simulink model "hyd_hyb_2_opt_cont_test_new_1_col_ef_fin_2"  (Simulink 7.7) (continued)
== system hyd_hyb_2_opt_cont_test_new_1_col_ef_fin_2/controller2/optimized controller/SOC upper 0.5/SOC upper 0.6/SOC under 0.7/net_col_e_f_6_7/Layer 2/LW{2,1} == (continued)
      mask Display: e = 0.06;c1 = [120 150 230]/255;c2 = ([120 150 230]+0)/383;c3 = ([120 150 230]+128)/383;patch([0 1 1 0],[0 0 1 1],c1);patch([0 1 1 1-e 1-e 0+e],[0 0 1 1-e 0+e 0+e],c2);patch([0 0 1 1-e 0+e 0+e],[0 1 1 1-e 1-e 0+e],c3);plot([0 1 1 0 0],[0 0 1 1 0]);disp('weights');
  "IW{2,1}(33,:)'" : Constant  Value=[-0.35234469279286512;-0.40468235895147475;-0.18096524442613818;-0.18151288248094333;0.35857480457232321;0.23465283463217285;0.40101555825099472;0.21139093219043778;-0.11912748111615507;0.29342558210195213;-0.15948625047030618;-0.11815965704262263;0.1116227020812456;0.38214609794411863;-0.35421490398026861;-0.27312335423238421;-0.26788573951818151;0.021395386612412425;-0.31654082740969164;-0.083409909154861142;-0.1468782051998643;0.21847941839715651;0.37294860709219418;0.2637687675371283;-0.074718043753328647;0.12745812216454461;-0.056240044727995646;0.22653279906164184;0.32679539257000695;0.33557761404320691;-0.01958919024617814;0.056572444135230458;0.30300666085920641;0.011579914144332524;0.26738390697574438;0.34894240758758227;-0.13804654423913723;0.13383865475945017;-0.21150677078154806;0.090005117551270664]
      mask Display: e = 0.06;c1 = [120 150 230]/255;c2 = ([120 150 230]+0)/383;c3 = ([120 150 230]+128)/383;patch([0 1 1 0],[0 0 1 1],c1);patch([0 1 1 1-e 1-e 0+e],[0 0 1 1-e 0+e 0+e],c2);patch([0 0 1 1-e 0+e 0+e],[0 1 1 1-e 1-e 0+e],c3);plot([0 1 1 0 0],[0 0 1 1 0]);disp('weights');
  "IW{2,1}(34,:)'" : Constant  Value=[0.15074995802727087;0.30864088922738236;0.10562014308619606;0.029979241891492325;-0.15584734818296847;-0.26021048893016635;-0.24865146505391678;0.41604231588507162;0.045896804265341991;-0.08694543014273505;-0.085027594542836857;0.45862186579974429;-0.31361816930303732;-0.12097477912022493;0.28844762834441939;0.066038996178467271;-0.068572896253808427;-0.09175843487790733;0.44634530134350081;0.17315383144725219;-0.29035181630262313;0.34479646540728387;-0.30962740604637629;-0.22996363447586768;-0.22948723755561082;0.31622260509068861;-0.25078044542389749;-0.37447691479609285;-0.35113036697226169;-0.089397504594286262;-0.255187761364293;0.079247556333326974;-0.20079103297549039;-0.074861134771812943;0.27127698682470541;0.38487777094434789;-0.2471333962217872;-0.089882718136514175;-0.20102325084023667;0.23002736531891085]
      mask Display: e = 0.06;c1 = [120 150 230]/255;c2 = ([120 150 230]+0)/383;c3 = ([120 150 230]+128)/383;patch([0 1 1 0],[0 0 1 1],c1);patch([0 1 1 1-e 1-e 0+e],[0 0 1 1-e 0+e 0+e],c2);patch([0 0 1 1-e 0+e 0+e],[0 1 1 1-e 1-e 0+e],c3);plot([0 1 1 0 0],[0 0 1 1 0]);disp('weights');
  "IW{2,1}(35,:)'" : Constant  Value=[-0.085280357024164019;-0.15814604519744255;-0.24621739793110575;0.43143057576690308;-0.42363845727206917;0.08687226022397769;0.21079716523428366;-0.024164079180984198;0.42797572604181494;0.4498416033934976;0.10822528990763226;0.085574245922645745;0.1522570064361313;-0.23438964187238326;0.2505811617273031;0.37801894202352326;-0.24299010034030982;0.019423098657966726;-0.10410706384354135;0.12024117541164461;0.37963413538941615;0.20515543726246629;-0.38789940580890198;0.10317756927625593;-0.36671922248120226;0.10963932782027253;0.074473878145015535;0.25249510438191625;0.022718384464952383;0.089004438179455844;-0.1812967994841308;-0.099778907647907422;-0.00013885403074624433;-0.35509553735099791;-0.02986583737910134;0.26514173132914987;0.41024463729816096;0.31064560212289161;0.22581215848793651;0.084557763920990112]
      mask Display: e = 0.06;c1 = [120 150 230]/255;c2 = ([120 150 230]+0)/383;c3 = ([120 150 230]+128)/383;patch([0 1 1 0],[0 0 1 1],c1);patch([0 1 1 1-e 1-e 0+e],[0 0 1 1-e 0+e 0+e],c2);patch([0 0 1 1-e 0+e 0+e],[0 1 1 1-e 1-e 0+e],c3);plot([0 1 1 0 0],[0 0 1 1 0]);disp('weights');
  "IW{2,1}(36,:)'" : Constant  Value=[0.11187917842212827;-0.15833534714410497;0.21442117909747105;0.18035201202815951;-0.20103443165034554;-0.43676294389703935;-0.24767795813336665;0.42859594714433347;-0.46594462872991904;0.38609488819476268;0.42804363407379792;-0.24835357807569508;0.35487975603085459;0.099382895784186964;0.037702915655006712;0.077359712591388144;-0.042553881876517007;0.22802448851846341;-0.15502215013149115;0.046833480077730839;-0.010626970787021696;-0.15587949850514998;0.10795274546066105;-0.35647469267790022;0.093489866349711181;-0.061913015016581703;0.0069346425814466352;0.23410829073547867;0.082823681928375095;0.084672792381860665;0.063329516746211914;0.22751356665680345;0.26551938770123051;-0.43991509625618636;-0.19202855444821165;0.1000567847980213;0.1197961732104279;-0.32733847522348158;0.36479375386410845;0.2732239191085889]
      mask Display: e = 0.06;c1 = [120 150 230]/255;c2 = ([120 150 230]+0)/383;c3 = ([120 150 230]+128)/383;patch([0 1 1 0],[0 0 1 1],c1);patch([0 1 1 1-e 1-e 0+e],[0 0 1 1-e 0+e 0+e],c2);patch([0 0 1 1-e 0+e 0+e],[0 1 1 1-e 1-e 0+e],c3);plot([0 1 1 0 0],[0 0 1 1 0]);disp('weights');
  "IW{2,1}(37,:)'" : Constant  Value=[0.22542906269712015;-0.23206687387491265;-0.41582441453719304;0.19907958152951435;-0.052390899388030987;-0.21713307410620905;-0.14279836338394636;-0.18034106153095997;0.38195893101400419;-0.20167227233343965;-0.10798221528183477;0.12776729738967238;0.12347559665720846;-0.39361066407783174;0.057989056419332705;-0.084862484010496314;-0.033437615546936063;-0.11558028023985598;0.021886547193143046;-0.34502232466084698;-0.3357414012306229;0.30873569567980058;-0.34857502847280747;-0.25620889903961552;0.14020136652308349;0.20416194799760654;-0.11813950443884963;0.27495061115104813;0.23013740973878341;-0.38843845697959822;-0.23459303861170966;0.29930194635965313;0.24309548875365156;0.256556613125552;0.41238162631707992;0.18772611001470427;-0.076315885440117276;0.012893512983791903;-0.43634903569871925;0.065732915816523241]
      mask Display: e = 0.06;c1 = [120 150 230]/255;c2 = ([120 150 230]+0)/383;c3 = ([120 150 230]+128)/383;patch([0 1 1 0],[0 0 1 1],c1);patch([0 1 1 1-e 1-e 0+e],[0 0 1 1-e 0+e 0+e],c2);patch([0 0 1 1-e 0+e 0+e],[0 1 1 1-e 1-e 0+e],c3);plot([0 1 1 0 0],[0 0 1 1 0]);disp('weights');
  "IW{2,1}(38,:)'" : Constant  Value=[-0.15687637927286577;0.36917633563686009;-0.29144238642137027;-0.28496243830489176;-0.29120265296463177;-0.16720472422586266;-0.32732491418049114;0.012380328221147003;0.25308608987500869;-0.31080168284313453;-0.14115897836824481;0.30823959639828868;0.13435007189910428;-0.27026968452106997;0.33353108706042345;-0.20586162066266542;0.35646268375518114;-0.29786664275900671;-0.037532512390921584;-0.23059109371293018;-0.32874031983206159;-0.065775359524783569;0.31255364238137584;0.13357880972227706;-0.32569441413025574;0.32777707861072408;-0.092206732296571184;0.12182727513782284;0.19694934568800054;-0.29716135192519855;0.12372996132474567;-0.29314142212795796;0.1007453511214564;0.12695639234602313;0.19962832326613919;-0.19600314744227701;-0.15468264750313671;0.27220846671165405;-0.36357404568227414;-0.22248043670160675]
      mask Display: e = 0.06;c1 = [120 150 230]/255;c2 = ([120 150 230]+0)/383;c3 = ([120 150 230]+128)/383;patch([0 1 1 0],[0 0 1 1],c1);patch([0 1 1 1-e 1-e 0+e],[0 0 1 1-e 0+e 0+e],c2);patch([0 0 1 1-e 0+e 0+e],[0 1 1 1-e 1-e 0+e],c3);plot([0 1 1 0 0],[0 0 1 1 0]);disp('weights');
  "IW{2,1}(39,:)'" : Constant  Value=[0.24058796492975254;0.3923243587570851;0.078966515972267892;-0.16394619579342773;-0.30280913050520986;0.3484741459001085;-0.12726912018809577;-0.22962738727879989;-0.04388378471388131;-0.23687844282218948;0.023563965371155793;-0.31482535629267333;-0.39420955887147413;-0.29577827442781379;-0.35103390143920965;0.063402770474746808;0.3284365627069033;0.1595091253598937;0.11070826187861493;-0.044032202526256531;0.36978057342829418;0.35721143459447674;0.16964431808255342;0.020192388082055583;-0.046017910286698605;0.402278478482547;0.11348150264565539;-0.43801214172903946;0.087002884619087589;0.071672830442686686;0.050469899960920474;-0.35116292344382749;-0.037997808720628551;0.14157546914372149;0.026100866768060997;-0.079742117838089863;0.08707088759100097;-0.36495123765462395;-0.35755801475726767;-0.26509645505088503]
      mask Display: e = 0.06;c1 = [120 150 230]/255;c2 = ([120 150 230]+0)/383;c3 = ([120 150 230]+128)/383;patch([0 1 1 0],[0 0 1 1],c1);patch([0 1 1 1-e 1-e 0+e],[0 0 1 1-e 0+e 0+e],c2);patch([0 0 1 1-e 0+e 0+e],[0 1 1 1-e 1-e 0+e],c3);plot([0 1 1 0 0],[0 0 1 1 0]);disp('weights');
  "IW{2,1}(4,:)'" : Constant  Value=[-0.01221140323552884;0.18072786298812832;-0.3140855165908864;-0.12099788973110417;-0.14523488288702574;-0.43700931256781916;0.42561098451762502;-0.21444295716308656;-0.41057632388322274;-0.37893180528552617;-0.031683877853917852;-0.23036116027979819;-0.16473141780181505;-0.35253387674590198;0.341963767625098;0.23912597139523462;0.30909431730506876;-0.36697578035509515;0.24200988103104415;-0.17643789536103216;-0.001019241624209845;0.29537860690834722;0.016094459145938728;-0.037543658468204864;-0.32564370819347827;-0.14949886992390565;0.0054613804968836827;-0.076340588784367183;-0.038168613201275396;0.26431828047945816;0.1920327419058856;-0.20127733354280752;-0.17784322199890351;0.1276306481919468;0.063327827863554592;0.13946038997141527;-0.12252141040949782;0.39836882652049344;-0.39764916157177288;-0.35786507616559005]
      mask Display: e = 0.06;c1 = [120 150 230]/255;c2 = ([120 150 230]+0)/383;c3 = ([120 150 230]+128)/383;patch([0 1 1 0],[0 0 1 1],c1);patch([0 1 1 1-e 1-e 0+e],[0 0 1 1-e 0+e 0+e],c2);patch([0 0 1 1-e 0+e 0+e],[0 1 1 1-e 1-e 0+e],c3);plot([0 1 1 0 0],[0 0 1 1 0]);disp('weights');
  "IW{2,1}(40,:)'" : Constant  Value=[-0.2977786014958943;0.18727182475970403;0.13467798845472928;0.076857101125664309;-0.18584526349064118;0.35512226754925064;-0.15661520913315033;-0.39811458268789479;0.38617345102519257;-0.17197958098024463;-0.34058983039534269;-0.13495905846225914;-0.18481750329056115;-0.08486830956154133;0.32310252648926008;-0.088847963238082611;-0.26900255736207407;0.42295876308626307;-0.16258324878140534;-0.075092546961337936;0.26431985915047473;-0.13978120836778424;-0.44168942945243578;0.14522828817594052;-0.081041133166638649;0.0034634335491892159;-0.084042534105895017;0.41568204161889105;0.31353846767808768;0.32386770769435796;-0.16174740047881567;0.26074364045686615;-0.16612768934716163;0.077983879794636363;0.15478651429146739;-0.35317869634842858;0.33259676584433623;-0.26549581598378302;-0.07896726765929496;-0.26229401937605112]
      mask Display: e = 0.06;c1 = [120 150 230]/255;c2 = ([120 150 230]+0)/383;c3 = ([120 150 230]+128)/383;patch([0 1 1 0],[0 0 1 1],c1);patch([0 1 1 1-e 1-e 0+e],[0 0 1 1-e 0+e 0+e],c2);patch([0 0 1 1-e 0+e 0+e],[0 1 1 1-e 1-e 0+e],c3);plot([0 1 1 0 0],[0 0 1 1 0]);disp('weights');
  "IW{2,1}(41,:)'" : Constant  Value=[0.12374463207755997;-0.28158655207815841;0.095589009979011041;-0.3235745029745532;-0.27310967972902223;0.22733011791120317;0.19831808496259232;0.00057962201351577597;0.071724425828926811;0.19048384458678946;0.36773949834236991;0.064053855217139147;-0.035613463718706634;-0.20216967365801861;-0.28007020467049937;0.24364774186005511;-0.1403404377486597;-0.050967598966734931;-0.16763395213635215;-0.20341622710789936;0.43084514323429318;-0.24361074047060993;-0.28677350264557416;0.14571519093268848;-0.23874680606219301;-0.25250218454533557;0.31533910494837741;0.38945443402948615;-0.13606527960694581;0.062069561913058138;0.088721912161191269;0.047018434001795527;-0.37942370927896968;-0.40725710006095339;-0.33740501650702037;0.2478864625186305;0.28386365132508418;-0.14097524665361313;0.23075320657061499;0.34003083768377967]
      mask Display: e = 0.06;c1 = [120 150 230]/255;c2 = ([120 150 230]+0)/383;c3 = ([120 150 230]+128)/383;patch([0 1 1 0],[0 0 1 1],c1);patch([0 1 1 1-e 1-e 0+e],[0 0 1 1-e 0+e 0+e],c2);patch([0 0 1 1-e 0+e 0+e],[0 1 1 1-e 1-e 0+e],c3);plot([0 1 1 0 0],[0 0 1 1 0]);disp('weights');
  "IW{2,1}(42,:)'" : Constant  Value=[-0.3505777576555813;-0.10999602423138424;0.30553694772179868;-0.10664651929504305;-0.30249080816352231;-0.13003161728713097;0.30683350520583913;0.41317558957586309;-0.30389328175577485;-0.21695178164448795;0.0031775306979481437;-0.012427164986180059;0.061179138449127923;0.23413387880260014;-0.11431185560742893;0.19921615325010597;0.28718734724044997;0.17696705069996821;-0.37706459191558217;-0.078774553000733369;-0.31208712531433175;-0.3569580615326558;0.22140312811492585;0.051341593285318773;0.32120033086946859;-0.030192407869492219;-0.14634065749356878;-0.26831791640789687;0.13749622178982507;-0.18708157332632702;-0.13830824842391906;-0.23059500983019787;-0.44154847323212926;-0.27873882384836446;0.28486587161326749;0.37593153678044644;0.42781288247884747;0.0032857441186048661;0.041166282396126533;-0.12426148756994582]
      mask Display: e = 0.06;c1 = [120 150 230]/255;c2 = ([120 150 230]+0)/383;c3 = ([120 150 230]+128)/383;patch([0 1 1 0],[0 0 1 1],c1);patch([0 1 1 1-e 1-e 0+e],[0 0 1 1-e 0+e 0+e],c2);patch([0 0 1 1-e 0+e 0+e],[0 1 1 1-e 1-e 0+e],c3);plot([0 1 1 0 0],[0 0 1 1 0]);disp('weights');
  "IW{2,1}(43,:)'" : Constant  Value=[-0.28284206118375033;-0.36311546878953466;0.30689587766983428;0.108845991936885;-0.24031228944312771;0.30154934077932222;-0.25412610019440668;-0.01918150170030811;0.28581766315806073;-0.10785434376307283;-0.25949570248524989;0.23479396205927056;-0.35613185960404459;-0.21607664044194339;-0.37283429390225165;0.23790879215982905;0.31868259314811448;-0.17765900646240576;0.30326262719508662;-0.045899657726886041;-0.31470971859168284;0.34019858096204886;-0.055034131947890023;-0.098124487040463532;-0.079524074549746346;0.24640470466577152;0.13875347903159121;-0.37489966207229808;0.2300931495036142;0.25528449654750995;0.12450861327235381;-0.13224675687262377;-0.32954454098584407;0.042449840898941077;0.35561545193798566;0.13425562374375302;-0.10150108311114024;-0.18589732358968489;-0.048415597487845657;-0.31802719225086684]
      mask Display: e = 0.06;c1 = [120 150 230]/255;c2 = ([120 150 230]+0)/383;c3 = ([120 150 230]+128)/383;patch([0 1 1 0],[0 0 1 1],c1);patch([0 1 1 1-e 1-e 0+e],[0 0 1 1-e 0+e 0+e],c2);patch([0 0 1 1-e 0+e 0+e],[0 1 1 1-e 1-e 0+e],c3);plot([0 1 1 0 0],[0 0 1 1 0]);disp('weights');
  "IW{2,1}(44,:)'" : Constant  Value=[0.24186756609886367;0.095626761068682481;0.26654656067729149;0.39643642757555436;-0.10425918489107079;-0.26391489579144017;-0.049893019446727367;-0.36958558815436737;-0.41536315585712119;-0.25940660039384267;0.28266445101991661;-0.37749239778290772;0.087014706296541219;-0.033609775651372818;-0.21665966922491384;0.06825768093279766;-0.40289599529495396;0.37876927109177572;-0.41873452944884437;0.24178274879595246;0.068391117677172486;0.095644761277350762;0.35331142901495649;0.058087080343530051;0.14037668371934481;0.20057470271574948;-0.28969763356565392;0.18991334315756131;0.071977111117728323;0.007847130043674537;0.2429287207714966;-0.24668758835818619;-0.19617478229712637;0.091426183332240937;-0.083169358743805169;-0.10413849932149064;0.27713246312576362;0.078656969478348909;0.36810666213474291;-0.30832420447422881]
      mask Display: e = 0.06;c1 = [120 150 230]/255;c2 = ([120 150 230]+0)/383;c3 = ([120 150 230]+128)/383;patch([0 1 1 0],[0 0 1 1],c1);patch([0 1 1 1-e 1-e 0+e],[0 0 1 1-e 0+e 0+e],c2);patch([0 0 1 1-e 0+e 0+e],[0 1 1 1-e 1-e 0+e],c3);plot([0 1 1 0 0],[0 0 1 1 0]);disp('weights');
  "IW{2,1}(45,:)'" : Constant  Value=[0.15801553328063686;0.2147950848714196;-0.10432151961439522;0.35287803443813565;-0.41349923728397608;0.12549140028135947;0.39922863113523932;-0.03498605999225167;-0.28755280948461548;-0.36499970043289315;-0.20358994128441979;-0.25782078255905444;0.011922789908669391;0.25532328024521378;-0.18723327739464099;0.28325370995158766;-0.25319754558012314;-0.23325080914514792;-0.066741860102844539;0.034476643291110803;0.14136647287229664;-0.27029024634068632;0.27454415669704146;-0.13749499473286073;0.31368285562019016;-0.12419702471524841;0.25200297985223702;0.39190773946821061;0.26454713274680408;-0.065334327629809924;-0.25503977586253629;0.0015780512603695012;0.36547133487036465;-0.42034430951905716;-0.13031134885640877;0.014551092701692156;0.39892420847612808;-0.17406942193360311;0.062344711607968943;-0.15053134249806502]
      mask Display: e = 0.06;c1 = [120 150 230]/255;c2 = ([120 150 230]+0)/383;c3 = ([120 150 230]+128)/383;patch([0 1 1 0],[0 0 1 1],c1);patch([0 1 1 1-e 1-e 0+e],[0 0 1 1-e 0+e 0+e],c2);patch([0 0 1 1-e 0+e 0+e],[0 1 1 1-e 1-e 0+e],c3);plot([0 1 1 0 0],[0 0 1 1 0]);disp('weights');
  "IW{2,1}(46,:)'" : Constant  Value=[-0.25346893568143747;0.11674230260896117;0.21247665319827722;-0.3023226316265718;0.40187215509063173;0.25179388244739503;-0.35482660278569744;-0.41651174379826861;-0.32396559297957056;0.077915869952318945;0.22280449623257417;0.10877322968338277;0.036141294556576831;-0.3485358781664159;0.32141807436619746;0.14854376989108528;0.10812867363128655;-0.26938446979961433;-0.25835872660066272;0.23251585870346667;0.40716747714170437;0.26793414586456421;-0.075165437384607334;0.29601224621827904;0.14197703179086099;-0.013831111239489052;-0.26813860878220697;0.34810237060033167;-0.28500503795060689;0.18951683840777356;-0.24261999984330401;0.10497856057091108;-0.012566490475294125;-0.16805804081586809;0.25540243616744723;0.31061497654270043;-0.27989029998371051;0.29625468641388025;-0.12489425730626975;0.012148501887610281]
      mask Display: e = 0.06;c1 = [120 150 230]/255;c2 = ([120 150 230]+0)/383;c3 = ([120 150 230]+128)/383;patch([0 1 1 0],[0 0 1 1],c1);patch([0 1 1 1-e 1-e 0+e],[0 0 1 1-e 0+e 0+e],c2);patch([0 0 1 1-e 0+e 0+e],[0 1 1 1-e 1-e 0+e],c3);plot([0 1 1 0 0],[0 0 1 1 0]);disp('weights');
  "IW{2,1}(47,:)'" : Constant  Value=[-0.16854743580021067;0.095087222038890029;0.24743230793525181;0.29113750231583885;0.33027710233818858;0.11584491491617656;0.31235286979033411;-0.25808866350084159;-0.22181754755204813;-0.20800748339377717;-0.27377489451822568;-0.18961470743893991;0.33024903069429828;0.09709101495274014;-0.19774033278608544;0.25622034772817109;-0.12761057645318893;-0.28207402884870236;0.32359854685351719;0.19148606877682869;0.1659100818996371;-0.22896322697040691;-0.11690933194391534;0.28206751525415219;0.19309652461392576;-0.32135572428465964;0.34436465561563601;0.25491020099889378;0.039812368894199222;-0.00045147899089443709;0.35801807198273933;-0.44150383595354553;-0.39280237255984529;-0.043673666389801344;-0.0044382786984080392;0.30094944022010744;0.17955990880744432;-0.11371270789555313;0.37299467764138;-0.042167474381799221]
      mask Display: e = 0.06;c1 = [120 150 230]/255;c2 = ([120 150 230]+0)/383;c3 = ([120 150 230]+128)/383;patch([0 1 1 0],[0 0 1 1],c1);patch([0 1 1 1-e 1-e 0+e],[0 0 1 1-e 0+e 0+e],c2);patch([0 0 1 1-e 0+e 0+e],[0 1 1 1-e 1-e 0+e],c3);plot([0 1 1 0 0],[0 0 1 1 0]);disp('weights');
  "IW{2,1}(48,:)'" : Constant  Value=[0.33580983684677068;-0.3557646378436905;-0.2609869867761912;0.41804217770025731;0.023018027558655944;0.30021868143595909;-0.21436857746172339;-0.33228020411141806;0.19401784216186033;0.18821683826027716;-0.29336978856191481;-0.29960260186473381;-0.079237285858170914;-0.10052576060754399;0.42546444450459026;-0.20530312551278571;-0.25493138728255849;-0.15575443563409963;-0.24795824142890097;0.21714853416644914;-0.17629417880485057;0.41521854751890536;-0.025068130748208971;0.078460162700272174;-0.22200641029381338;-0.14732513315524082;0.084248299372936583;-0.066942842273487735;-0.018727673723746188;0.25505570248698373;-0.26857039064822424;-0.16545383905599903;-0.17576286045151351;-0.33753246371969065;0.27704733533396336;0.11682621568904474;-0.34026989373512978;0.022581869684723314;-0.3851876763316629;0.10156330710757971]
      mask Display: e = 0.06;c1 = [120 150 230]/255;c2 = ([120 150 230]+0)/383;c3 = ([120 150 230]+128)/383;patch([0 1 1 0],[0 0 1 1],c1);patch([0 1 1 1-e 1-e 0+e],[0 0 1 1-e 0+e 0+e],c2);patch([0 0 1 1-e 0+e 0+e],[0 1 1 1-e 1-e 0+e],c3);plot([0 1 1 0 0],[0 0 1 1 0]);disp('weights');
  "IW{2,1}(49,:)'" : Constant  Value=[-0.33847322256086276;0.18399516477309463;-0.23473724108862554;0.069633550031784425;0.10998955394256775;0.0038943158929632018;-0.26467106671932872;0.17057712141887085;0.40790150453658502;-0.3350429444319758;-0.34754441102565686;-0.13779475241325068;0.01918018770690259;0.27109047390203661;0.046727451342470416;0.20206169358839687;0.055715676298250624;0.43431386643861192;-0.27705620142915849;-0.1819380346905943;-0.26804847135279319;0.41459019776243788;0.35624877493101592;-0.22297365719943338;0.16592070646605131;0.074935196503958815;0.098118676524609358;-0.092153900905186978;-0.010018666586662681;-0.40674001339342669;-0.092167022925548112;0.20750098759103347;0.27631682681232994;-0.25558249934564159;-0.17075704022289051;0.1308954452526549;-0.38469168342270949;-0.019230167025044431;-0.16684428615676411;-0.17105092650225021]
      mask Display: e = 0.06;c1 = [120 150 230]/255;c2 = ([120 150 230]+0)/383;c3 = ([120 150 230]+128)/383;patch([0 1 1 0],[0 0 1 1],c1);patch([0 1 1 1-e 1-e 0+e],[0 0 1 1-e 0+e 0+e],c2);patch([0 0 1 1-e 0+e 0+e],[0 1 1 1-e 1-e 0+e],c3);plot([0 1 1 0 0],[0 0 1 1 0]);disp('weights');
  "IW{2,1}(5,:)'" : Constant  Value=[-0.043667973291560608;-0.19317481198184952;0.26061427287101585;0.081418532731955512;-0.029854039038156848;0.17215255969961135;0.0095169198179909809;0.22464710725917864;0.034611270072751971;0.12162500155145399;0.26703195378681799;-0.39207552206692275;-0.43337327250492247;-0.18890260306526221;-0.27925846232824864;-0.17460496219465627;-0.33581971389463844;-0.062847775390875341;0.18535730826764527;-0.39905784764506175;0.1617405149581338;0.054106271889717124;0.36331286012046932;0.2887209661180149;0.14601191414494805;0.29186319507277136;0.12667346920099506;0.37853400027901618;-0.0052462852625326101;0.45140719124070905;-0.43974328396455753;0.10262024303283142;-0.020368275419533632;-0.28212267577199246;-0.35888146315328923;0.020218125050021813;0.083137800400369527;0.063340354492734133;0.06527211215727606;0.30090077398017984]
      mask Display: e = 0.06;c1 = [120 150 230]/255;c2 = ([120 150 230]+0)/383;c3 = ([120 150 230]+128)/383;patch([0 1 1 0],[0 0 1 1],c1);patch([0 1 1 1-e 1-e 0+e],[0 0 1 1-e 0+e 0+e],c2);patch([0 0 1 1-e 0+e 0+e],[0 1 1 1-e 1-e 0+e],c3);plot([0 1 1 0 0],[0 0 1 1 0]);disp('weights');
  "IW{2,1}(50,:)'" : Constant  Value=[0.36088224772749156;0.14454564693499183;-0.076837639620770684;0.27692136809642243;-0.22835335472073534;-0.10683327100455513;-0.16826337418608692;0.22903102211681817;0.38509443582084341;0.35064259529936215;-0.17983861644941293;0.21238280913351903;0.042565499312524062;-0.26046958680244048;-0.034309890280299939;-0.32364731021950333;-0.0051258238978388682;-0.34062840148964829;0.29280496134516215;0.3302665558495958;0.13694089800654391;0.18294879569155206;0.40218883585512671;-0.19325983417104117;-0.042717035493806788;-0.45873393194075185;-0.15441445846299459;0.053049904764862092;0.41036108486011424;0.31723394985761727;0.30299169645771679;0.081457498534366971;0.14640619125825677;-0.25990835486823372;-0.33356624955022013;-0.15505198323550667;0.22819507796591793;-0.099056901810265302;0.088478546574233208;-0.34981641403767516]
      mask Display: e = 0.06;c1 = [120 150 230]/255;c2 = ([120 150 230]+0)/383;c3 = ([120 150 230]+128)/383;patch([0 1 1 0],[0 0 1 1],c1);patch([0 1 1 1-e 1-e 0+e],[0 0 1 1-e 0+e 0+e],c2);patch([0 0 1 1-e 0+e 0+e],[0 1 1 1-e 1-e 0+e],c3);plot([0 1 1 0 0],[0 0 1 1 0]);disp('weights');
  "IW{2,1}(6,:)'" : Constant  Value=[0.050737487410425178;0.33697864039954312;0.11508820994787418;0.33052765249423371;-0.28715239840786633;0.15145507097912184;-0.16516742551558031;0.44416723087952931;0.19439508783029597;0.35866936111782316;0.37954238599984447;-0.23627356612277706;-0.26864592776412816;0.27289341286878055;-0.29181832610913977;-0.33627650968285844;0.42031522032429669;0.0099350670051968903;0.026786340650303803;0.21467256398318849;-0.18396184449749395;-0.38073150085455931;-0.10051809274792767;0.13976783706552387;0.22969782998647839;0.19936564506397539;-0.23201717988110207;0.0015818442126015702;0.42949531070711044;-0.084642291458395677;-0.087096743709423802;0.19867617956133474;-0.0052857865714212278;0.1069699523921372;0.16071948054418889;0.23556452421303758;-0.18607220623058593;-0.35181324456593255;-0.27728586605665378;-0.028489040420667171]
      mask Display: e = 0.06;c1 = [120 150 230]/255;c2 = ([120 150 230]+0)/383;c3 = ([120 150 230]+128)/383;patch([0 1 1 0],[0 0 1 1],c1);patch([0 1 1 1-e 1-e 0+e],[0 0 1 1-e 0+e 0+e],c2);patch([0 0 1 1-e 0+e 0+e],[0 1 1 1-e 1-e 0+e],c3);plot([0 1 1 0 0],[0 0 1 1 0]);disp('weights');
  "IW{2,1}(7,:)'" : Constant  Value=[-0.15783057888941771;0.074944307425545961;-0.010669118037353332;0.23971451441184463;-0.24763078786340978;0.055016467812084968;-0.0059983444243396607;-0.37079551784857306;-0.49310921691804738;-0.31696677895664527;0.20628793226048761;0.17002319067569419;0.17784699529145087;-0.11598918041795532;-0.00465617470742758;0.25129390922924572;-0.20366702428986064;0.10184590478261686;0.12239850745139623;-0.27273312202462296;0.00084849036327700887;0.0063692769663586052;0.21419829878677479;-0.46186966293344101;-0.14849026634011242;0.2861084899133377;-0.35654854599279623;0.17120748228534907;-0.45354305225425845;-0.069393077792743077;0.30929869872242405;0.023451057498884483;0.27773444659765772;0.45288727871132056;-0.058783727694184065;-0.39145472460645003;-0.35377844731042507;-0.1825440283915219;0.12108323179725025;-0.086311398589953126]
      mask Display: e = 0.06;c1 = [120 150 230]/255;c2 = ([120 150 230]+0)/383;c3 = ([120 150 230]+128)/383;patch([0 1 1 0],[0 0 1 1],c1);patch([0 1 1 1-e 1-e 0+e],[0 0 1 1-e 0+e 0+e],c2);patch([0 0 1 1-e 0+e 0+e],[0 1 1 1-e 1-e 0+e],c3);plot([0 1 1 0 0],[0 0 1 1 0]);disp('weights');
  "IW{2,1}(8,:)'" : Constant  Value=[-0.10644766622345599;0.30143481403056471;0.34553909834029523;0.0018684493395994617;0.19684399635818667;0.1322749271963429;0.13332392876085764;-0.18759449204135831;-0.12676955296155334;0.056954991726560454;0.16714790830805645;-0.11298184132561552;-0.13536611280361169;-0.024925962676348917;0.44439726365526871;-0.26143694835101322;0.010852245784042371;0.41649355354343193;0.021871204814493863;0.22346667624066227;-0.060871472568119624;0.14470449224820545;-0.13854678872608275;0.3242095442487678;-0.064282547801060019;-0.41546090131535474;-0.014654634033789528;0.38680280797599553;0.2638379859340233;-0.30280261517527468;-0.32530725966142399;0.49796672606167536;0.11050078918378926;-0.3762906410885114;0.32968902143519008;0.22680014365067672;-0.25340687194442629;-0.28681137831293019;-0.058212228141198766;0.41923429030360171]
      mask Display: e = 0.06;c1 = [120 150 230]/255;c2 = ([120 150 230]+0)/383;c3 = ([120 150 230]+128)/383;patch([0 1 1 0],[0 0 1 1],c1);patch([0 1 1 1-e 1-e 0+e],[0 0 1 1-e 0+e 0+e],c2);patch([0 0 1 1-e 0+e 0+e],[0 1 1 1-e 1-e 0+e],c3);plot([0 1 1 0 0],[0 0 1 1 0]);disp('weights');
  "IW{2,1}(9,:)'" : Constant  Value=[0.20746308944798442;0.29797991913509414;0.076777573901370813;-0.11792061209779846;-0.13207032642050603;0.31052811061821534;-0.43469254603055291;0.20224865335496575;0.44498852837107405;0.17711089871136007;0.014692002878479252;0.079517102509166077;-0.31795399500078037;0.30883707114134035;-0.20267702199217794;-0.24727532544746902;0.013020539082115493;0.023105390115368028;0.11904573994523757;0.39971423510228371;0.25726149250009317;-0.072948748608184774;-0.092759568957091698;0.38151942860722077;-0.27573396789183402;0.18178959065247804;-0.31121086105740836;-0.43323963814014765;0.14019279884023605;0.028048843052972491;-0.16805117507261044;-0.29487878917708094;0.29317260840566389;-0.0050319996592048388;0.42698184199327871;-0.28184337027482131;0.023911208316360372;0.044284870971817145;0.21523655973032599;0.084888330817957269]
      mask Display: e = 0.06;c1 = [120 150 230]/255;c2 = ([120 150 230]+0)/383;c3 = ([120 150 230]+128)/383;patch([0 1 1 0],[0 0 1 1],c1);patch([0 1 1 1-e 1-e 0+e],[0 0 1 1-e 0+e 0+e],c2);patch([0 0 1 1-e 0+e 0+e],[0 1 1 1-e 1-e 0+e],c3);plot([0 1 1 0 0],[0 0 1 1 0]);disp('weights');
  Mux : Mux  Inputs=50
      mask Display: e = 0.06;c1 = [120 150 230]/255;c2 = ([120 150 230]+0)/383;c3 = ([120 150 230]+128)/383;patch([0 1 1 0],[0 0 1 1],c1);patch([0 1 1 1-e 1-e 0+e],[0 0 1 1-e 0+e 0+e],c2);patch([0 0 1 1-e 0+e 0+e],[0 1 1 1-e 1-e 0+e],c3);plot([0 1 1 0 0],[0 0 1 1 0]);disp('Mux');
  dotprod{1..50} (50 alike) : neural/Weight Functions/dotprod  SystemSampleTime=-1
  "lz{2,1}" : Outport
      mask Display: e = 0.06;c1 = [120 230 180]/255;c2 = ([120 230 180]+0)/(256+128);c3 = ([120 230 180]+128)/(256+128);patch([0 1 1 0],[0 0 1 1],c1);patch([0 1 1 1-e 1-e 0+e],[0 0 1 1-e 0+e 0+e],c2);patch([0 0 1 1-e 0+e 0+e],[0 1 1 1-e 1-e 0+e],c3);plot([0 1 1 0 0],[0 0 1 1 0]);
  wires:
  Mux/1 -> "lz{2,1}"/1
  dotprod50/1 -> Mux/50
  "IW{2,1}(50,:)'"/1 -> dotprod50/1
  dotprod49/1 -> Mux/49
  "IW{2,1}(49,:)'"/1 -> dotprod49/1
  dotprod48/1 -> Mux/48
  "IW{2,1}(48,:)'"/1 -> dotprod48/1
  dotprod47/1 -> Mux/47
  "IW{2,1}(47,:)'"/1 -> dotprod47/1
  dotprod46/1 -> Mux/46
  "IW{2,1}(46,:)'"/1 -> dotprod46/1
  dotprod45/1 -> Mux/45
  "IW{2,1}(45,:)'"/1 -> dotprod45/1
  dotprod44/1 -> Mux/44
  "IW{2,1}(44,:)'"/1 -> dotprod44/1
  dotprod43/1 -> Mux/43
  "IW{2,1}(43,:)'"/1 -> dotprod43/1
  dotprod42/1 -> Mux/42
  "IW{2,1}(42,:)'"/1 -> dotprod42/1
  dotprod41/1 -> Mux/41
  "IW{2,1}(41,:)'"/1 -> dotprod41/1
  dotprod40/1 -> Mux/40
  "IW{2,1}(40,:)'"/1 -> dotprod40/1
  dotprod39/1 -> Mux/39
  "IW{2,1}(39,:)'"/1 -> dotprod39/1
  dotprod38/1 -> Mux/38
  "IW{2,1}(38,:)'"/1 -> dotprod38/1
  dotprod37/1 -> Mux/37
  "IW{2,1}(37,:)'"/1 -> dotprod37/1
  dotprod36/1 -> Mux/36
  "IW{2,1}(36,:)'"/1 -> dotprod36/1
  dotprod35/1 -> Mux/35
  "IW{2,1}(35,:)'"/1 -> dotprod35/1
  dotprod34/1 -> Mux/34
  "IW{2,1}(34,:)'"/1 -> dotprod34/1
  dotprod33/1 -> Mux/33
  "IW{2,1}(33,:)'"/1 -> dotprod33/1
  dotprod32/1 -> Mux/32
  "IW{2,1}(32,:)'"/1 -> dotprod32/1
  dotprod31/1 -> Mux/31
  "IW{2,1}(31,:)'"/1 -> dotprod31/1
  dotprod30/1 -> Mux/30
  "IW{2,1}(30,:)'"/1 -> dotprod30/1
  dotprod29/1 -> Mux/29
  "IW{2,1}(29,:)'"/1 -> dotprod29/1
  dotprod28/1 -> Mux/28
  "IW{2,1}(28,:)'"/1 -> dotprod28/1
  dotprod27/1 -> Mux/27
  "IW{2,1}(27,:)'"/1 -> dotprod27/1
  dotprod26/1 -> Mux/26
  "IW{2,1}(26,:)'"/1 -> dotprod26/1
  dotprod25/1 -> Mux/25
  "IW{2,1}(25,:)'"/1 -> dotprod25/1
  dotprod24/1 -> Mux/24
  "IW{2,1}(24,:)'"/1 -> dotprod24/1
  dotprod23/1 -> Mux/23
  "IW{2,1}(23,:)'"/1 -> dotprod23/1
  dotprod22/1 -> Mux/22
  "IW{2,1}(22,:)'"/1 -> dotprod22/1
  dotprod21/1 -> Mux/21
  "IW{2,1}(21,:)'"/1 -> dotprod21/1
  dotprod20/1 -> Mux/20
  "IW{2,1}(20,:)'"/1 -> dotprod20/1
  dotprod19/1 -> Mux/19
  "IW{2,1}(19,:)'"/1 -> dotprod19/1
  dotprod18/1 -> Mux/18
  "IW{2,1}(18,:)'"/1 -> dotprod18/1
  dotprod17/1 -> Mux/17
  "IW{2,1}(17,:)'"/1 -> dotprod17/1
  dotprod16/1 -> Mux/16
  "IW{2,1}(16,:)'"/1 -> dotprod16/1
  dotprod15/1 -> Mux/15
  "IW{2,1}(15,:)'"/1 -> dotprod15/1
  dotprod14/1 -> Mux/14
  "IW{2,1}(14,:)'"/1 -> dotprod14/1
  dotprod13/1 -> Mux/13
  "IW{2,1}(13,:)'"/1 -> dotprod13/1
  dotprod12/1 -> Mux/12
  "IW{2,1}(12,:)'"/1 -> dotprod12/1
  dotprod11/1 -> Mux/11
  "IW{2,1}(11,:)'"/1 -> dotprod11/1
  dotprod10/1 -> Mux/10
  "IW{2,1}(10,:)'"/1 -> dotprod10/1
  dotprod9/1 -> Mux/9
  "IW{2,1}(9,:)'"/1 -> dotprod9/1
  dotprod8/1 -> Mux/8
  "IW{2,1}(8,:)'"/1 -> dotprod8/1
  dotprod7/1 -> Mux/7
  "IW{2,1}(7,:)'"/1 -> dotprod7/1
  dotprod6/1 -> Mux/6
  "IW{2,1}(6,:)'"/1 -> dotprod6/1
  dotprod5/1 -> Mux/5
  "IW{2,1}(5,:)'"/1 -> dotprod5/1
  dotprod4/1 -> Mux/4
  "IW{2,1}(4,:)'"/1 -> dotprod4/1
  dotprod3/1 -> Mux/3
  "IW{2,1}(3,:)'"/1 -> dotprod3/1
  dotprod2/1 -> Mux/2
  "IW{2,1}(2,:)'"/1 -> dotprod2/1
  dotprod1/1 -> Mux/1
  "ad{2,1}"/1 -> dotprod50/2, dotprod49/2, dotprod48/2, dotprod47/2, dotprod46/2, dotprod45/2, dotprod44/2, dotprod43/2, dotprod42/2, dotprod41/2, dotprod40/2, dotprod39/2, dotprod38/2, dotprod37/2, dotprod36/2, dotprod35/2, dotprod34/2, dotprod33/2, dotprod32/2, dotprod31/2, dotprod30/2, dotprod29/2, dotprod28/2, dotprod27/2, dotprod26/2, dotprod25/2, dotprod24/2, dotprod23/2, dotprod22/2, dotprod21/2, dotprod20/2, dotprod19/2, dotprod18/2, dotprod17/2, dotprod16/2, dotprod15/2, dotprod14/2, dotprod13/2, dotprod12/2, dotprod11/2, dotprod10/2, dotprod9/2, dotprod8/2, dotprod7/2, dotprod6/2, dotprod5/2, dotprod4/2, dotprod3/2, dotprod2/2, dotprod1/2
  "IW{2,1}(1,:)'"/1 -> dotprod1/1
